simulink model "CCMVgaMotor"  (R2022b)
settings: Solver=FixedStepDiscrete  StartTime=0.0  StopTime=10.0  FixedStep=0.01  RelTol=1e-3
model workspace (MAT data in the model file):
  NvMBlockVgaMtrCalData : Simulink.Signal (value not decoded)
  NvMBlockVgaMtrCalDataInit : Simulink.Parameter (value not decoded)
  VgaWakeUpFlag : Simulink.Signal (value not decoded)
symbols that are objects in the model workspace (value not decoded): NvMBlockVgaMtrCalDataInit
undefined symbols (not defined in the file or in its folder): ComChlType, DioChannelType, LinFrameType, NetworkChlType, NvMBlockId, P_DioLevelType, P_LinIfSchHandleType, P_Std_ReturnType, PowerChlType, VGAChlType
(part 3 of 4)

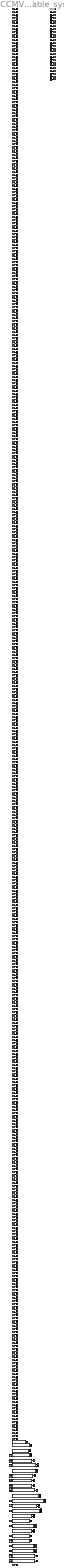
== system CCMVgaMotor/CCMVgaMotor_Runnable_sys ==
  CarCfgForClimaCtrl_CarCfgForAirClng_read : Inport
  CarCfgForClimaCtrl_CarCfgForAirClnrAdv_read : Inport  Port=2
  CarCfgForClimaCtrl_CarCfgForAirFrag_read : Inport  Port=3
  CarCfgForClimaCtrl_CarCfgForAirMtrlSnsr_read : Inport  Port=4
  CarCfgForClimaCtrl_CarCfgForAirQly_read : Inport  Port=5
  CarCfgForClimaCtrl_CarCfgForAmntOfSeat_read : Inport  Port=6
  CarCfgForClimaCtrl_CarCfgForAuxWtrPmp_read : Inport  Port=7
  CarCfgForClimaCtrl_CarCfgForBypVlvInHvac_read : Inport  Port=8
  CarCfgForClimaCtrl_CarCfgForClsVlvInHvac_read : Inport  Port=9
  CarCfgForClimaCtrl_CarCfgForCmprAcTyp_read : Inport  Port=10
  CarCfgForClimaCtrl_CarCfgForCmptmtClng_read : Inport  Port=11
  CarCfgForClimaCtrl_CarCfgForElecVentAdjmt_read : Inport  Port=12
  CarCfgForClimaCtrl_CarCfgForFlcTyp_read : Inport  Port=13
  CarCfgForClimaCtrl_CarCfgForFoldCSD_read : Inport  Port=14
  CarCfgForClimaCtrl_CarCfgForFuTyp_read : Inport  Port=15
  CarCfgForClimaCtrl_CarCfgForGlassTyp_read : Inport  Port=16
  CarCfgForClimaCtrl_CarCfgForHeatPmp_read : Inport  Port=17
  CarCfgForClimaCtrl_CarCfgForHeatrDurgDrvg_read : Inport  Port=18
  CarCfgForClimaCtrl_CarCfgForHeatrTyp_read : Inport  Port=19
  CarCfgForClimaCtrl_CarCfgForHvacFilTyp_read : Inport  Port=20
  CarCfgForClimaCtrl_CarCfgForHvacFlrSnsrForT_read : Inport  Port=21
  CarCfgForClimaCtrl_CarCfgForHvacHeatrSnsrT_read : Inport  Port=22
  CarCfgForClimaCtrl_CarCfgForHvacRe_read : Inport  Port=23
  CarCfgForClimaCtrl_CarCfgForHvacTyp_read : Inport  Port=24
  CarCfgForClimaCtrl_CarCfgForLoVoltElecCfg_read : Inport  Port=25
  CarCfgForClimaCtrl_CarCfgForNavSysandIntnmap_read : Inport  Port=26
  CarCfgForClimaCtrl_CarCfgForNrOfDoor_read : Inport  Port=27
  CarCfgForClimaCtrl_CarCfgForPipeInFuTank_read : Inport  Port=28
  CarCfgForClimaCtrl_CarCfgForPreClng_read : Inport  Port=29
  CarCfgForClimaCtrl_CarCfgForPrkgCDSNotAvl_read : Inport  Port=30
  CarCfgForClimaCtrl_CarCfgForPrkgClima_read : Inport  Port=31
  CarCfgForClimaCtrl_CarCfgForPrpsnTyp_read : Inport  Port=32
  CarCfgForClimaCtrl_CarCfgForRemEngStrtPreCdn_read : Inport  Port=33
  CarCfgForClimaCtrl_CarCfgForRoofHatch_read : Inport  Port=34
  CarCfgForClimaCtrl_CarCfgForSeatHeatgCal_read : Inport  Port=35
  CarCfgForClimaCtrl_CarCfgForSeatHeatgForRowFirst_read : Inport  Port=36
  CarCfgForClimaCtrl_CarCfgForSeatHeatgForRowSec_read : Inport  Port=37
  CarCfgForClimaCtrl_CarCfgForSeatVentnForRowFirst_read : Inport  Port=38
  CarCfgForClimaCtrl_CarCfgForSeatVentnForRowSec_read : Inport  Port=39
  CarCfgForClimaCtrl_CarCfgForSecRowVentnCenter_read : Inport  Port=40
  CarCfgForClimaCtrl_CarCfgForSecRowVentnSide_read : Inport  Port=41
  CarCfgForClimaCtrl_CarCfgForSmallFlowCyc_read : Inport  Port=42
  CarCfgForClimaCtrl_CarCfgForSmkSnsr_read : Inport  Port=43
  CarCfgForClimaCtrl_CarCfgForSnsrForCmptmtT_read : Inport  Port=44
  CarCfgForClimaCtrl_CarCfgForSnsrHumPrsnt_read : Inport  Port=45
  CarCfgForClimaCtrl_CarCfgForSteerWhlHeatg_read : Inport  Port=46
  CarCfgForClimaCtrl_CarCfgForSteerWhlPosn_read : Inport  Port=47
  CarCfgForClimaCtrl_CarCfgForSunSnsr_read : Inport  Port=48
  CarCfgForClimaCtrl_CarCfgForTelmNodTyp_read : Inport  Port=49
  CarCfgForClimaCtrl_CarCfgForThermMngt_read : Inport  Port=50
  CarCfgForClimaCtrl_CarCfgForVehTyp_read : Inport  Port=51
  CarCfgForClimaCtrl_CarCfgForVgaMotorExistSts_read : Inport  Port=52
  CarCfgForClimaCtrl_CarCfgForWindHeatd_read : Inport  Port=53
  CarCfgForClimaCtrl_CarCfgForAutDemst_read : Inport  Port=54
  CarCfgForClimaCtrl_CarCfgForExtVentnClimaCtrl_read : Inport  Port=55
  CarCfgForClimaCtrl_CarCfgForStartDevice_read : Inport  Port=56
  HmiElecAirSwngReq_HmiElecAirSwngReq_read : Inport  Port=57
  LIN2ScheTableReq_Val_read : Inport  Port=58
  SysServCarCfgSts_SysServCarID_read : Inport  Port=59
  SysServCarCfgSts_SysServCCPAQSSts_read : Inport  Port=60
  SysServCarCfgSts_SysServCCPHEXSensorSts_read : Inport  Port=61
  SysServCarCfgSts_SysServCCPLin2AFUSts_read : Inport  Port=62
  SysServCarCfgSts_SysServCCPLin2AIUSts_read : Inport  Port=63
  SysServCarCfgSts_SysServCCPLin2CDSSts_read : Inport  Port=64
  SysServCarCfgSts_SysServCCPLin2PMSISts_read : Inport  Port=65
  SysServCarCfgSts_SysServCCPLin3VGASts_read : Inport  Port=66
  SysServCarCfgSts_SysServDUAirVentSnrSts_read : Inport  Port=67
  SysServCarCfgSts_SysServDUBlowerPwmSignSts_read : Inport  Port=68
  SysServCarCfgSts_SysServDUBlowerPwmSts_read : Inport  Port=69
  SysServCarCfgSts_SysServDUDCDefrostSts_read : Inport  Port=70
  SysServCarCfgSts_SysServDUDCModeSts_read : Inport  Port=71
  SysServCarCfgSts_SysServDUDCRecSts_read : Inport  Port=72
  SysServCarCfgSts_SysServDUDCSModeSts_read : Inport  Port=73
  SysServCarCfgSts_SysServDUDCSTLSts_read : Inport  Port=74
  SysServCarCfgSts_SysServDUDCTLSts_read : Inport  Port=75
  SysServCarCfgSts_SysServDUDCTRSts_read : Inport  Port=76
  SysServCarCfgSts_SysServDUFLFootSts_read : Inport  Port=77
  SysServCarCfgSts_SysServDUFLVentSts_read : Inport  Port=78
  SysServCarCfgSts_SysServDUFRFootSts_read : Inport  Port=79
  SysServCarCfgSts_SysServDUFRVentSts_read : Inport  Port=80
  SysServCarCfgSts_SysServDUIncarMotorSts_read : Inport  Port=81
  SysServCarCfgSts_SysServDULin1HBMFSts_read : Inport  Port=82
  SysServCarCfgSts_SysServDULin4HFAFSts_read : Inport  Port=83
  SysServCarCfgSts_SysServDUSFLFootSts_read : Inport  Port=84
  SysServCarCfgSts_SysServDUSFLVentSts_read : Inport  Port=85
  SysServCarCfgSts_SysServDUSFRFootSts_read : Inport  Port=86
  SysServCarCfgSts_SysServDUSFRVentSts_read : Inport  Port=87
  SysServCarCfgSts_SysServDUSnsrForFCmptmtTSts_read : Inport  Port=88
  SysServCarCfgSts_SysServDUSnsrForRair_read : Inport  Port=89
  SysServCarCfgSts_SysServDUSnsrForRCmptmtTSts_read : Inport  Port=90
  VehModMngtGlbSafe1_VehModMngtGlbSafe1_read : Inport  Port=91
  BatLoadBtrMSts_BatLoadBtrMSts_read : Inport  Port=92
  BatSysBtrMSts_BatSysBtrMSts_read : Inport  Port=93
  VgaMotSetPowerOnInit_PowerOnInit_read : Inport  Port=94
  CarCfgForClimaCtrl_CarCfgForBRAND_read : Inport  Port=95
  CarCfgForClimaCtrl_CarCfgForHeadRadar_read : Inport  Port=96
  CarCfgForClimaCtrl_CarCfgForInteriorViewCamera_read : Inport  Port=97
  CarCfgForClimaCtrl_CarCfgForLifeDetection_read : Inport  Port=98
  CarCfgForClimaCtrl_CarCfgForPowerSeats_read : Inport  Port=99
  CarCfgForClimaCtrl_CarCfgForPTCInFrontHVAC_read : Inport  Port=100
  CarCfgForClimaCtrl_CarCfgForVentLiftMotorExiSts_read : Inport  Port=101
  CarCfgForClimaCtrl_CarCfgForWadingAssistSystem_read : Inport  Port=102
  SbcPwrOn_SbcPwrOn_read : Inport  Port=103
  SbcWakeupSta_SbcWakeupSta_read : Inport  Port=104
  SysServCarCfgSts_SysServCCPBGMNum_read : Inport  Port=105
  SysServCarCfgSts_SysServCCPLin2AQSSts_read : Inport  Port=106
  SysServCarCfgSts_SysServDUBlowerPwmSignTypeSts_read : Inport  Port=107
  SysServCarCfgSts_SysServDUDCFLMdeSts_read : Inport  Port=108
  SysServCarCfgSts_SysServDUReBlowerPwmSignSts_read : Inport  Port=109
  SysServCarCfgSts_SysServDUReBlowerPwmSts_read : Inport  Port=110
  SysServCarCfgSts_SysServLowSideRETXVSts_read : Inport  Port=111
  VentnActr01ActPson_VentnActr01ActPson_read : Inport  Port=112
  VentnActr01ActPson_VentnActr01ActPson_IsUpdated_read : Inport  Port=113
  VentnActr01BlckInd_VentnActr01BlckInd_read : Inport  Port=114
  VentnActr01BlckSts_VentnActr01BlckSts_read : Inport  Port=115
  VentnActr01BusAdr_VentnActr01BusAdr_read : Inport  Port=116
  VentnActr01CoilSts_VentnActr01CoilSts_read : Inport  Port=117
  VentnActr01DrvDir_VentnActr01DrvDir_read : Inport  Port=118
  VentnActr01ElecErr_VentnActr01ElecErr_read : Inport  Port=119
  VentnActr01Mode_VentnActr01Mode_read : Inport  Port=120
  VentnActr01MovDir_VentnActr01MovDir_read : Inport  Port=121
  VentnActr01OvrTempErr_VentnActr01OvrTempErr_read : Inport  Port=122
  VentnActr01PsonSts_VentnActr01PsonSts_read : Inport  Port=123
  VentnActr01ResetSts_VentnActr01ResetSts_read : Inport  Port=124
  VentnActr01SpclFct_VentnActr01SpclFct_read : Inport  Port=125
  VentnActr01SpdSts_VentnActr01SpdSts_read : Inport  Port=126
  VentnActr01TrqSts_VentnActr01TrqSts_read : Inport  Port=127
  VentnActr01UrgcExist_VentnActr01UrgcExist_read : Inport  Port=128
  VentnActr01UrgcPson_VentnActr01UrgcPson_read : Inport  Port=129
  VentnActr01UrgcSts_VentnActr01UrgcSts_read : Inport  Port=130
  VentnActr01VoltErr_VentnActr01VoltErr_read : Inport  Port=131
  VentnActr02ActPson_VentnActr02ActPson_read : Inport  Port=132
  VentnActr02ActPson_VentnActr02ActPson_IsUpdated_read : Inport  Port=133
  VentnActr02BlckInd_VentnActr02BlckInd_read : Inport  Port=134
  VentnActr02BlckSts_VentnActr02BlckSts_read : Inport  Port=135
  VentnActr02BusAdr_VentnActr02BusAdr_read : Inport  Port=136
  VentnActr02CoilSts_VentnActr02CoilSts_read : Inport  Port=137
  VentnActr02DrvDir_VentnActr02DrvDir_read : Inport  Port=138
  VentnActr02ElecErr_VentnActr02ElecErr_read : Inport  Port=139
  VentnActr02Mode_VentnActr02Mode_read : Inport  Port=140
  VentnActr02MovDir_VentnActr02MovDir_read : Inport  Port=141
  VentnActr02OvrTempErr_VentnActr02OvrTempErr_read : Inport  Port=142
  VentnActr02PsonSts_VentnActr02PsonSts_read : Inport  Port=143
  VentnActr02ResetSts_VentnActr02ResetSts_read : Inport  Port=144
  VentnActr02SpclFct_VentnActr02SpclFct_read : Inport  Port=145
  VentnActr02SpdSts_VentnActr02SpdSts_read : Inport  Port=146
  VentnActr02TrqSts_VentnActr02TrqSts_read : Inport  Port=147
  VentnActr02UrgcExist_VentnActr02UrgcExist_read : Inport  Port=148
  VentnActr02UrgcPson_VentnActr02UrgcPson_read : Inport  Port=149
  VentnActr02UrgcSts_VentnActr02UrgcSts_read : Inport  Port=150
  VentnActr02VoltErr_VentnActr02VoltErr_read : Inport  Port=151
  VentnActr03ActPson_VentnActr03ActPson_read : Inport  Port=152
  VentnActr03ActPson_VentnActr03ActPson_IsUpdated_read : Inport  Port=153
  VentnActr03BlckInd_VentnActr03BlckInd_read : Inport  Port=154
  VentnActr03BlckSts_VentnActr03BlckSts_read : Inport  Port=155
  VentnActr03BusAdr_VentnActr03BusAdr_read : Inport  Port=156
  VentnActr03CoilSts_VentnActr03CoilSts_read : Inport  Port=157
  VentnActr03DrvDir_VentnActr03DrvDir_read : Inport  Port=158
  VentnActr03ElecErr_VentnActr03ElecErr_read : Inport  Port=159
  VentnActr03Mode_VentnActr03Mode_read : Inport  Port=160
  VentnActr03MovDir_VentnActr03MovDir_read : Inport  Port=161
  VentnActr03OvrTempErr_VentnActr03OvrTempErr_read : Inport  Port=162
  VentnActr03PsonSts_VentnActr03PsonSts_read : Inport  Port=163
  VentnActr03ResetSts_VentnActr03ResetSts_read : Inport  Port=164
  VentnActr03SpclFct_VentnActr03SpclFct_read : Inport  Port=165
  VentnActr03SpdSts_VentnActr03SpdSts_read : Inport  Port=166
  VentnActr03TrqSts_VentnActr03TrqSts_read : Inport  Port=167
  VentnActr03UrgcExist_VentnActr03UrgcExist_read : Inport  Port=168
  VentnActr03UrgcPson_VentnActr03UrgcPson_read : Inport  Port=169
  VentnActr03UrgcSts_VentnActr03UrgcSts_read : Inport  Port=170
  VentnActr03VoltErr_VentnActr03VoltErr_read : Inport  Port=171
  VentnActr04ActPson_VentnActr04ActPson_read : Inport  Port=172
  VentnActr04ActPson_VentnActr04ActPson_IsUpdated_read : Inport  Port=173
  VentnActr04BlckInd_VentnActr04BlckInd_read : Inport  Port=174
  VentnActr04BlckSts_VentnActr04BlckSts_read : Inport  Port=175
  VentnActr04BusAdr_VentnActr04BusAdr_read : Inport  Port=176
  VentnActr04CoilSts_VentnActr04CoilSts_read : Inport  Port=177
  VentnActr04DrvDir_VentnActr04DrvDir_read : Inport  Port=178
  VentnActr04ElecErr_VentnActr04ElecErr_read : Inport  Port=179
  VentnActr04Mode_VentnActr04Mode_read : Inport  Port=180
  VentnActr04MovDir_VentnActr04MovDir_read : Inport  Port=181
  VentnActr04OvrTempErr_VentnActr04OvrTempErr_read : Inport  Port=182
  VentnActr04PsonSts_VentnActr04PsonSts_read : Inport  Port=183
  VentnActr04ResetSts_VentnActr04ResetSts_read : Inport  Port=184
  VentnActr04SpclFct_VentnActr04SpclFct_read : Inport  Port=185
  VentnActr04SpdSts_VentnActr04SpdSts_read : Inport  Port=186
  VentnActr04TrqSts_VentnActr04TrqSts_read : Inport  Port=187
  VentnActr04UrgcExist_VentnActr04UrgcExist_read : Inport  Port=188
  VentnActr04UrgcPson_VentnActr04UrgcPson_read : Inport  Port=189
  VentnActr04UrgcSts_VentnActr04UrgcSts_read : Inport  Port=190
  VentnActr04VoltErr_VentnActr04VoltErr_read : Inport  Port=191
  VentnActr05ActPson_VentnActr05ActPson_read : Inport  Port=192
  VentnActr05ActPson_VentnActr05ActPson_IsUpdated_read : Inport  Port=193
  VentnActr05BlckInd_VentnActr05BlckInd_read : Inport  Port=194
  VentnActr05BlckSts_VentnActr05BlckSts_read : Inport  Port=195
  VentnActr05BusAdr_VentnActr05BusAdr_read : Inport  Port=196
  VentnActr05CoilSts_VentnActr05CoilSts_read : Inport  Port=197
  VentnActr05DrvDir_VentnActr05DrvDir_read : Inport  Port=198
  VentnActr05ElecErr_VentnActr05ElecErr_read : Inport  Port=199
  VentnActr05Mode_VentnActr05Mode_read : Inport  Port=200
  VentnActr05MovDir_VentnActr05MovDir_read : Inport  Port=201
  VentnActr05OvrTempErr_VentnActr05OvrTempErr_read : Inport  Port=202
  VentnActr05PsonSts_VentnActr05PsonSts_read : Inport  Port=203
  VentnActr05ResetSts_VentnActr05ResetSts_read : Inport  Port=204
  VentnActr05SpclFct_VentnActr05SpclFct_read : Inport  Port=205
  VentnActr05SpdSts_VentnActr05SpdSts_read : Inport  Port=206
  VentnActr05TrqSts_VentnActr05TrqSts_read : Inport  Port=207
  VentnActr05UrgcExist_VentnActr05UrgcExist_read : Inport  Port=208
  VentnActr05UrgcPson_VentnActr05UrgcPson_read : Inport  Port=209
  VentnActr05UrgcSts_VentnActr05UrgcSts_read : Inport  Port=210
  VentnActr05VoltErr_VentnActr05VoltErr_read : Inport  Port=211
  VentnActr06ActPson_VentnActr06ActPson_read : Inport  Port=212
  VentnActr06ActPson_VentnActr06ActPson_IsUpdated_read : Inport  Port=213
  VentnActr06BlckInd_VentnActr06BlckInd_read : Inport  Port=214
  VentnActr06BlckSts_VentnActr06BlckSts_read : Inport  Port=215
  VentnActr06BusAdr_VentnActr06BusAdr_read : Inport  Port=216
  VentnActr06CoilSts_VentnActr06CoilSts_read : Inport  Port=217
  VentnActr06DrvDir_VentnActr06DrvDir_read : Inport  Port=218
  VentnActr06ElecErr_VentnActr06ElecErr_read : Inport  Port=219
  VentnActr06Mode_VentnActr06Mode_read : Inport  Port=220
  VentnActr06MovDir_VentnActr06MovDir_read : Inport  Port=221
  VentnActr06OvrTempErr_VentnActr06OvrTempErr_read : Inport  Port=222
  VentnActr06PsonSts_VentnActr06PsonSts_read : Inport  Port=223
  VentnActr06ResetSts_VentnActr06ResetSts_read : Inport  Port=224
  VentnActr06SpclFct_VentnActr06SpclFct_read : Inport  Port=225
  VentnActr06SpdSts_VentnActr06SpdSts_read : Inport  Port=226
  VentnActr06TrqSts_VentnActr06TrqSts_read : Inport  Port=227
  VentnActr06UrgcExist_VentnActr06UrgcExist_read : Inport  Port=228
  VentnActr06UrgcPson_VentnActr06UrgcPson_read : Inport  Port=229
  VentnActr06UrgcSts_VentnActr06UrgcSts_read : Inport  Port=230
  VentnActr06VoltErr_VentnActr06VoltErr_read : Inport  Port=231
  VentnActr07ActPson_VentnActr07ActPson_read : Inport  Port=232
  VentnActr07ActPson_VentnActr07ActPson_IsUpdated_read : Inport  Port=233
  VentnActr07BlckInd_VentnActr07BlckInd_read : Inport  Port=234
  VentnActr07BlckSts_VentnActr07BlckSts_read : Inport  Port=235
  VentnActr07BusAdr_VentnActr07BusAdr_read : Inport  Port=236
  VentnActr07CoilSts_VentnActr07CoilSts_read : Inport  Port=237
  VentnActr07DrvDir_VentnActr07DrvDir_read : Inport  Port=238
  VentnActr07ElecErr_VentnActr07ElecErr_read : Inport  Port=239
  VentnActr07Mode_VentnActr07Mode_read : Inport  Port=240
  VentnActr07MovDir_VentnActr07MovDir_read : Inport  Port=241
  VentnActr07OvrTempErr_VentnActr07OvrTempErr_read : Inport  Port=242
  VentnActr07PsonSts_VentnActr07PsonSts_read : Inport  Port=243
  VentnActr07ResetSts_VentnActr07ResetSts_read : Inport  Port=244
  VentnActr07SpclFct_VentnActr07SpclFct_read : Inport  Port=245
  VentnActr07SpdSts_VentnActr07SpdSts_read : Inport  Port=246
  VentnActr07TrqSts_VentnActr07TrqSts_read : Inport  Port=247
  VentnActr07UrgcExist_VentnActr07UrgcExist_read : Inport  Port=248
  VentnActr07UrgcPson_VentnActr07UrgcPson_read : Inport  Port=249
  VentnActr07UrgcSts_VentnActr07UrgcSts_read : Inport  Port=250
  VentnActr07VoltErr_VentnActr07VoltErr_read : Inport  Port=251
  VentnActr08ActPson_VentnActr08ActPson_read : Inport  Port=252
  VentnActr08ActPson_VentnActr08ActPson_IsUpdated_read : Inport  Port=253
  VentnActr08BlckInd_VentnActr08BlckInd_read : Inport  Port=254
  VentnActr08BlckSts_VentnActr08BlckSts_read : Inport  Port=255
  VentnActr08BusAdr_VentnActr08BusAdr_read : Inport  Port=256
  VentnActr08CoilSts_VentnActr08CoilSts_read : Inport  Port=257
  VentnActr08DrvDir_VentnActr08DrvDir_read : Inport  Port=258
  VentnActr08ElecErr_VentnActr08ElecErr_read : Inport  Port=259
  VentnActr08Mode_VentnActr08Mode_read : Inport  Port=260
  VentnActr08MovDir_VentnActr08MovDir_read : Inport  Port=261
  VentnActr08OvrTempErr_VentnActr08OvrTempErr_read : Inport  Port=262
  VentnActr08PsonSts_VentnActr08PsonSts_read : Inport  Port=263
  VentnActr08ResetSts_VentnActr08ResetSts_read : Inport  Port=264
  VentnActr08SpclFct_VentnActr08SpclFct_read : Inport  Port=265
  VentnActr08SpdSts_VentnActr08SpdSts_read : Inport  Port=266
  VentnActr08TrqSts_VentnActr08TrqSts_read : Inport  Port=267
  VentnActr08UrgcExist_VentnActr08UrgcExist_read : Inport  Port=268
  VentnActr08UrgcPson_VentnActr08UrgcPson_read : Inport  Port=269
  VentnActr08UrgcSts_VentnActr08UrgcSts_read : Inport  Port=270
  VentnActr08VoltErr_VentnActr08VoltErr_read : Inport  Port=271
  VentnActr09ActPson_VentnActr09ActPson_read : Inport  Port=272
  VentnActr09ActPson_VentnActr09ActPson_IsUpdated_read : Inport  Port=273
  VentnActr09BlckInd_VentnActr09BlckInd_read : Inport  Port=274
  VentnActr09BlckSts_VentnActr09BlckSts_read : Inport  Port=275
  VentnActr09BusAdr_VentnActr09BusAdr_read : Inport  Port=276
  VentnActr09CoilSts_VentnActr09CoilSts_read : Inport  Port=277
  VentnActr09DrvDir_VentnActr09DrvDir_read : Inport  Port=278
  VentnActr09ElecErr_VentnActr09ElecErr_read : Inport  Port=279
  VentnActr09Mode_VentnActr09Mode_read : Inport  Port=280
  VentnActr09MovDir_VentnActr09MovDir_read : Inport  Port=281
  VentnActr09OvrTempErr_VentnActr09OvrTempErr_read : Inport  Port=282
  VentnActr09PsonSts_VentnActr09PsonSts_read : Inport  Port=283
  VentnActr09ResetSts_VentnActr09ResetSts_read : Inport  Port=284
  VentnActr09SpclFct_VentnActr09SpclFct_read : Inport  Port=285
  VentnActr09SpdSts_VentnActr09SpdSts_read : Inport  Port=286
  VentnActr09TrqSts_VentnActr09TrqSts_read : Inport  Port=287
  VentnActr09UrgcExist_VentnActr09UrgcExist_read : Inport  Port=288
  VentnActr09UrgcPson_VentnActr09UrgcPson_read : Inport  Port=289
  VentnActr09UrgcSts_VentnActr09UrgcSts_read : Inport  Port=290
  VentnActr09VoltErr_VentnActr09VoltErr_read : Inport  Port=291
  VentnActr10ActPson_VentnActr10ActPson_read : Inport  Port=292
  VentnActr10ActPson_VentnActr10ActPson_IsUpdated_read : Inport  Port=293
  VentnActr10BlckInd_VentnActr10BlckInd_read : Inport  Port=294
  VentnActr10BlckSts_VentnActr10BlckSts_read : Inport  Port=295
  VentnActr10BusAdr_VentnActr10BusAdr_read : Inport  Port=296
  VentnActr10CoilSts_VentnActr10CoilSts_read : Inport  Port=297
  VentnActr10DrvDir_VentnActr10DrvDir_read : Inport  Port=298
  VentnActr10ElecErr_VentnActr10ElecErr_read : Inport  Port=299
  VentnActr10Mode_VentnActr10Mode_read : Inport  Port=300
  VentnActr10MovDir_VentnActr10MovDir_read : Inport  Port=301
  VentnActr10OvrTempErr_VentnActr10OvrTempErr_read : Inport  Port=302
  VentnActr10PsonSts_VentnActr10PsonSts_read : Inport  Port=303
  VentnActr10ResetSts_VentnActr10ResetSts_read : Inport  Port=304
  VentnActr10SpclFct_VentnActr10SpclFct_read : Inport  Port=305
  VentnActr10SpdSts_VentnActr10SpdSts_read : Inport  Port=306
  VentnActr10TrqSts_VentnActr10TrqSts_read : Inport  Port=307
  VentnActr10UrgcExist_VentnActr10UrgcExist_read : Inport  Port=308
  VentnActr10UrgcPson_VentnActr10UrgcPson_read : Inport  Port=309
  VentnActr10UrgcSts_VentnActr10UrgcSts_read : Inport  Port=310
  VentnActr10VoltErr_VentnActr10VoltErr_read : Inport  Port=311
  VentnActr11ActPson_VentnActr11ActPson_read : Inport  Port=312
  VentnActr11ActPson_VentnActr11ActPson_IsUpdated_read : Inport  Port=313
  VentnActr11BlckInd_VentnActr11BlckInd_read : Inport  Port=314
  VentnActr11BlckSts_VentnActr11BlckSts_read : Inport  Port=315
  VentnActr11BusAdr_VentnActr11BusAdr_read : Inport  Port=316
  VentnActr11CoilSts_VentnActr11CoilSts_read : Inport  Port=317
  VentnActr11DrvDir_VentnActr11DrvDir_read : Inport  Port=318
  VentnActr11ElecErr_VentnActr11ElecErr_read : Inport  Port=319
  VentnActr11Mode_VentnActr11Mode_read : Inport  Port=320
  VentnActr11MovDir_VentnActr11MovDir_read : Inport  Port=321
  VentnActr11OvrTempErr_VentnActr11OvrTempErr_read : Inport  Port=322
  VentnActr11PsonSts_VentnActr11PsonSts_read : Inport  Port=323
  VentnActr11ResetSts_VentnActr11ResetSts_read : Inport  Port=324
  VentnActr11SpclFct_VentnActr11SpclFct_read : Inport  Port=325
  VentnActr11SpdSts_VentnActr11SpdSts_read : Inport  Port=326
  VentnActr11TrqSts_VentnActr11TrqSts_read : Inport  Port=327
  VentnActr11UrgcExist_VentnActr11UrgcExist_read : Inport  Port=328
  VentnActr11UrgcPson_VentnActr11UrgcPson_read : Inport  Port=329
  VentnActr11UrgcSts_VentnActr11UrgcSts_read : Inport  Port=330
  VentnActr11VoltErr_VentnActr11VoltErr_read : Inport  Port=331
  VentnActr12ActPson_VentnActr12ActPson_read : Inport  Port=332
  VentnActr12ActPson_VentnActr12ActPson_IsUpdated_read : Inport  Port=333
  VentnActr12BlckInd_VentnActr12BlckInd_read : Inport  Port=334
  VentnActr12BlckSts_VentnActr12BlckSts_read : Inport  Port=335
  VentnActr12BusAdr_VentnActr12BusAdr_read : Inport  Port=336
  VentnActr12CoilSts_VentnActr12CoilSts_read : Inport  Port=337
  VentnActr12DrvDir_VentnActr12DrvDir_read : Inport  Port=338
  VentnActr12ElecErr_VentnActr12ElecErr_read : Inport  Port=339
  VentnActr12Mode_VentnActr12Mode_read : Inport  Port=340
  VentnActr12MovDir_VentnActr12MovDir_read : Inport  Port=341
  VentnActr12OvrTempErr_VentnActr12OvrTempErr_read : Inport  Port=342
  VentnActr12PsonSts_VentnActr12PsonSts_read : Inport  Port=343
  VentnActr12ResetSts_VentnActr12ResetSts_read : Inport  Port=344
  VentnActr12SpclFct_VentnActr12SpclFct_read : Inport  Port=345
  VentnActr12SpdSts_VentnActr12SpdSts_read : Inport  Port=346
  VentnActr12TrqSts_VentnActr12TrqSts_read : Inport  Port=347
  VentnActr12UrgcExist_VentnActr12UrgcExist_read : Inport  Port=348
  VentnActr12UrgcPson_VentnActr12UrgcPson_read : Inport  Port=349
  VentnActr12UrgcSts_VentnActr12UrgcSts_read : Inport  Port=350
  VentnActr12VoltErr_VentnActr12VoltErr_read : Inport  Port=351
  VentnActr13ActPson_VentnActr13ActPson_read : Inport  Port=352
  VentnActr13ActPson_VentnActr13ActPson_IsUpdated_read : Inport  Port=353
  VentnActr13BlckInd_VentnActr13BlckInd_read : Inport  Port=354
  VentnActr13BlckSts_VentnActr13BlckSts_read : Inport  Port=355
  VentnActr13BusAdr_VentnActr13BusAdr_read : Inport  Port=356
  VentnActr13CoilSts_VentnActr13CoilSts_read : Inport  Port=357
  VentnActr13DrvDir_VentnActr13DrvDir_read : Inport  Port=358
  VentnActr13ElecErr_VentnActr13ElecErr_read : Inport  Port=359
  VentnActr13Mode_VentnActr13Mode_read : Inport  Port=360
  VentnActr13MovDir_VentnActr13MovDir_read : Inport  Port=361
  VentnActr13OvrTempErr_VentnActr13OvrTempErr_read : Inport  Port=362
  VentnActr13PsonSts_VentnActr13PsonSts_read : Inport  Port=363
  VentnActr13ResetSts_VentnActr13ResetSts_read : Inport  Port=364
  VentnActr13SpclFct_VentnActr13SpclFct_read : Inport  Port=365
  VentnActr13SpdSts_VentnActr13SpdSts_read : Inport  Port=366
  VentnActr13TrqSts_VentnActr13TrqSts_read : Inport  Port=367
  VentnActr13UrgcExist_VentnActr13UrgcExist_read : Inport  Port=368
  VentnActr13UrgcPson_VentnActr13UrgcPson_read : Inport  Port=369
  VentnActr13UrgcSts_VentnActr13UrgcSts_read : Inport  Port=370
  VentnActr13VoltErr_VentnActr13VoltErr_read : Inport  Port=371
  VentnActr14ActPson_VentnActr14ActPson_read : Inport  Port=372
  VentnActr14ActPson_VentnActr14ActPson_IsUpdated_read : Inport  Port=373
  VentnActr14BlckInd_VentnActr14BlckInd_read : Inport  Port=374
  VentnActr14BlckSts_VentnActr14BlckSts_read : Inport  Port=375
  VentnActr14BusAdr_VentnActr14BusAdr_read : Inport  Port=376
  VentnActr14CoilSts_VentnActr14CoilSts_read : Inport  Port=377
  VentnActr14DrvDir_VentnActr14DrvDir_read : Inport  Port=378
  VentnActr14ElecErr_VentnActr14ElecErr_read : Inport  Port=379
  VentnActr14Mode_VentnActr14Mode_read : Inport  Port=380
  VentnActr14MovDir_VentnActr14MovDir_read : Inport  Port=381
  VentnActr14OvrTempErr_VentnActr14OvrTempErr_read : Inport  Port=382
  VentnActr14PsonSts_VentnActr14PsonSts_read : Inport  Port=383
  VentnActr14ResetSts_VentnActr14ResetSts_read : Inport  Port=384
  VentnActr14SpclFct_VentnActr14SpclFct_read : Inport  Port=385
  VentnActr14SpdSts_VentnActr14SpdSts_read : Inport  Port=386
  VentnActr14TrqSts_VentnActr14TrqSts_read : Inport  Port=387
  VentnActr14UrgcExist_VentnActr14UrgcExist_read : Inport  Port=388
  VentnActr14UrgcPson_VentnActr14UrgcPson_read : Inport  Port=389
  VentnActr14UrgcSts_VentnActr14UrgcSts_read : Inport  Port=390
  VentnActr14VoltErr_VentnActr14VoltErr_read : Inport  Port=391
  VentnActr15ActPson_VentnActr15ActPson_read : Inport  Port=392
  VentnActr15ActPson_VentnActr15ActPson_IsUpdated_read : Inport  Port=393
  VentnActr15BlckInd_VentnActr15BlckInd_read : Inport  Port=394
  VentnActr15BlckSts_VentnActr15BlckSts_read : Inport  Port=395
  VentnActr15BusAdr_VentnActr15BusAdr_read : Inport  Port=396
  VentnActr15CoilSts_VentnActr15CoilSts_read : Inport  Port=397
  VentnActr15DrvDir_VentnActr15DrvDir_read : Inport  Port=398
  VentnActr15ElecErr_VentnActr15ElecErr_read : Inport  Port=399
  VentnActr15Mode_VentnActr15Mode_read : Inport  Port=400
  VentnActr15MovDir_VentnActr15MovDir_read : Inport  Port=401
  VentnActr15OvrTempErr_VentnActr15OvrTempErr_read : Inport  Port=402
  VentnActr15PsonSts_VentnActr15PsonSts_read : Inport  Port=403
  VentnActr15ResetSts_VentnActr15ResetSts_read : Inport  Port=404
  VentnActr15SpclFct_VentnActr15SpclFct_read : Inport  Port=405
  VentnActr15SpdSts_VentnActr15SpdSts_read : Inport  Port=406
  VentnActr15TrqSts_VentnActr15TrqSts_read : Inport  Port=407
  VentnActr15UrgcExist_VentnActr15UrgcExist_read : Inport  Port=408
  VentnActr15UrgcPson_VentnActr15UrgcPson_read : Inport  Port=409
  VentnActr15UrgcSts_VentnActr15UrgcSts_read : Inport  Port=410
  VentnActr15VoltErr_VentnActr15VoltErr_read : Inport  Port=411
  VentnActr16ActPson_VentnActr16ActPson_read : Inport  Port=412
  VentnActr16BlckInd_VentnActr16BlckInd_read : Inport  Port=413
  VentnActr16BlckSts_VentnActr16BlckSts_read : Inport  Port=414
  VentnActr16BusAdr_VentnActr16BusAdr_read : Inport  Port=415
  VentnActr16CoilSts_VentnActr16CoilSts_read : Inport  Port=416
  VentnActr16DrvDir_VentnActr16DrvDir_read : Inport  Port=417
  VentnActr16ElecErr_VentnActr16ElecErr_read : Inport  Port=418
  VentnActr16Mode_VentnActr16Mode_read : Inport  Port=419
  VentnActr16MovDir_VentnActr16MovDir_read : Inport  Port=420
  VentnActr16OvrTempErr_VentnActr16OvrTempErr_read : Inport  Port=421
  VentnActr16PsonSts_VentnActr16PsonSts_read : Inport  Port=422
  VentnActr16ResetSts_VentnActr16ResetSts_read : Inport  Port=423
  VentnActr16SpclFct_VentnActr16SpclFct_read : Inport  Port=424
  VentnActr16SpdSts_VentnActr16SpdSts_read : Inport  Port=425
  VentnActr16TrqSts_VentnActr16TrqSts_read : Inport  Port=426
  VentnActr16UrgcExist_VentnActr16UrgcExist_read : Inport  Port=427
  VentnActr16UrgcPson_VentnActr16UrgcPson_read : Inport  Port=428
  VentnActr16UrgcSts_VentnActr16UrgcSts_read : Inport  Port=429
  VentnActr16VoltErr_VentnActr16VoltErr_read : Inport  Port=430
  VentnActr17ActPson_VentnActr17ActPson_read : Inport  Port=431
  VentnActr17ActPson_VentnActr17ActPson_IsUpdated_read : Inport  Port=432
  VentnActr17BlckInd_VentnActr17BlckInd_read : Inport  Port=433
  VentnActr17BlckSts_VentnActr17BlckSts_read : Inport  Port=434
  VentnActr17BusAdr_VentnActr17BusAdr_read : Inport  Port=435
  VentnActr17CoilSts_VentnActr17CoilSts_read : Inport  Port=436
  VentnActr17DrvDir_VentnActr17DrvDir_read : Inport  Port=437
  VentnActr17ElecErr_VentnActr17ElecErr_read : Inport  Port=438
  VentnActr17Mode_VentnActr17Mode_read : Inport  Port=439
  VentnActr17MovDir_VentnActr17MovDir_read : Inport  Port=440
  VentnActr17OvrTempErr_VentnActr17OvrTempErr_read : Inport  Port=441
  VentnActr17PsonSts_VentnActr17PsonSts_read : Inport  Port=442
  VentnActr17ResetSts_VentnActr17ResetSts_read : Inport  Port=443
  VentnActr17SpclFct_VentnActr17SpclFct_read : Inport  Port=444
  VentnActr17SpdSts_VentnActr17SpdSts_read : Inport  Port=445
  VentnActr17TrqSts_VentnActr17TrqSts_read : Inport  Port=446
  VentnActr17UrgcExist_VentnActr17UrgcExist_read : Inport  Port=447
  VentnActr17UrgcPson_VentnActr17UrgcPson_read : Inport  Port=448
  VentnActr17UrgcSts_VentnActr17UrgcSts_read : Inport  Port=449
  VentnActr17VoltErr_VentnActr17VoltErr_read : Inport  Port=450
  VentnActr18ActPson_VentnActr18ActPson_read : Inport  Port=451
  VentnActr18BlckInd_VentnActr18BlckInd_read : Inport  Port=452
  VentnActr18BlckSts_VentnActr18BlckSts_read : Inport  Port=453
  VentnActr18BusAdr_VentnActr18BusAdr_read : Inport  Port=454
  VentnActr18CoilSts_VentnActr18CoilSts_read : Inport  Port=455
  VentnActr18DrvDir_VentnActr18DrvDir_read : Inport  Port=456
  VentnActr18ElecErr_VentnActr18ElecErr_read : Inport  Port=457
  VentnActr18Mode_VentnActr18Mode_read : Inport  Port=458
  VentnActr18MovDir_VentnActr18MovDir_read : Inport  Port=459
  VentnActr18OvrTempErr_VentnActr18OvrTempErr_read : Inport  Port=460
  VentnActr18PsonSts_VentnActr18PsonSts_read : Inport  Port=461
  VentnActr18ResetSts_VentnActr18ResetSts_read : Inport  Port=462
  VentnActr18SpclFct_VentnActr18SpclFct_read : Inport  Port=463
  VentnActr18SpdSts_VentnActr18SpdSts_read : Inport  Port=464
  VentnActr18TrqSts_VentnActr18TrqSts_read : Inport  Port=465
  VentnActr18UrgcExist_VentnActr18UrgcExist_read : Inport  Port=466
  VentnActr18UrgcPson_VentnActr18UrgcPson_read : Inport  Port=467
  VentnActr18UrgcSts_VentnActr18UrgcSts_read : Inport  Port=468
  VentnActr18VoltErr_VentnActr18VoltErr_read : Inport  Port=469
  VentnActr19ActPson_VentnActr19ActPson_read : Inport  Port=470
  VentnActr19ActPson_VentnActr19ActPson_IsUpdated_read : Inport  Port=471
  VentnActr19BlckInd_VentnActr19BlckInd_read : Inport  Port=472
  VentnActr19BlckSts_VentnActr19BlckSts_read : Inport  Port=473
  VentnActr19BusAdr_VentnActr19BusAdr_read : Inport  Port=474
  VentnActr19CoilSts_VentnActr19CoilSts_read : Inport  Port=475
  VentnActr19DrvDir_VentnActr19DrvDir_read : Inport  Port=476
  VentnActr19ElecErr_VentnActr19ElecErr_read : Inport  Port=477
  VentnActr19Mode_VentnActr19Mode_read : Inport  Port=478
  VentnActr19MovDir_VentnActr19MovDir_read : Inport  Port=479
  VentnActr19OvrTempErr_VentnActr19OvrTempErr_read : Inport  Port=480
  VentnActr19PsonSts_VentnActr19PsonSts_read : Inport  Port=481
  VentnActr19ResetSts_VentnActr19ResetSts_read : Inport  Port=482
  VentnActr19SpclFct_VentnActr19SpclFct_read : Inport  Port=483
  VentnActr19SpdSts_VentnActr19SpdSts_read : Inport  Port=484
  VentnActr19TrqSts_VentnActr19TrqSts_read : Inport  Port=485
  VentnActr19UrgcExist_VentnActr19UrgcExist_read : Inport  Port=486
  VentnActr19UrgcPson_VentnActr19UrgcPson_read : Inport  Port=487
  VentnActr19UrgcSts_VentnActr19UrgcSts_read : Inport  Port=488
  VentnActr19VoltErr_VentnActr19VoltErr_read : Inport  Port=489
  VentnActr20ActPson_VentnActr20ActPson_read : Inport  Port=490
  VentnActr20ActPson_VentnActr20ActPson_IsUpdated_read : Inport  Port=491
  VentnActr20BlckInd_VentnActr20BlckInd_read : Inport  Port=492
  VentnActr20BlckSts_VentnActr20BlckSts_read : Inport  Port=493
  VentnActr20BusAdr_VentnActr20BusAdr_read : Inport  Port=494
  VentnActr20CoilSts_VentnActr20CoilSts_read : Inport  Port=495
  VentnActr20DrvDir_VentnActr20DrvDir_read : Inport  Port=496
  VentnActr20ElecErr_VentnActr20ElecErr_read : Inport  Port=497
  VentnActr20Mode_VentnActr20Mode_read : Inport  Port=498
  VentnActr20MovDir_VentnActr20MovDir_read : Inport  Port=499
  VentnActr20OvrTempErr_VentnActr20OvrTempErr_read : Inport  Port=500
  VentnActr20PsonSts_VentnActr20PsonSts_read : Inport  Port=501
  VentnActr20ResetSts_VentnActr20ResetSts_read : Inport  Port=502
  VentnActr20SpclFct_VentnActr20SpclFct_read : Inport  Port=503
  VentnActr20SpdSts_VentnActr20SpdSts_read : Inport  Port=504
  VentnActr20TrqSts_VentnActr20TrqSts_read : Inport  Port=505
  VentnActr20UrgcExist_VentnActr20UrgcExist_read : Inport  Port=506
  VentnActr20UrgcPson_VentnActr20UrgcPson_read : Inport  Port=507
  VentnActr20UrgcSts_VentnActr20UrgcSts_read : Inport  Port=508
  VentnActr20VoltErr_VentnActr20VoltErr_read : Inport  Port=509
  VGAStopDuePowerLimit_VGAStopDuePowerLimit_read : Inport  Port=510
  BswLifeCycle_LifeCycle_read : Inport  Port=511
  function : TriggerPort  TriggerType=function-call  VariantControl=(inherit)  SampleTimeType=periodic  SampleTime=-1
  DioServ_PowerOffInitChannel : FunctionCaller  FunctionPrototype=ERR = DioServ_PowerOffInitChannel()  OutputArgumentSpecifications=P_Std_ReturnType
  DioServ_PowerOnInitChannel : FunctionCaller  FunctionPrototype=ERR = DioServ_PowerOnInitChannel()  OutputArgumentSpecifications=P_Std_ReturnType
  DioServ_ReadChannel : FunctionCaller  FunctionPrototype=[Level,ERR] = DioServ_ReadChannel(ChannelId)  InputArgumentSpecifications=DioChannelType(0)  OutputArgumentSpecifications=P_DioLevelType, P_Std_ReturnType
  DioServ_WriteChannel : FunctionCaller  FunctionPrototype=ERR = DioServ_WriteChannel(ChannelId,Level)  InputArgumentSpecifications=DioChannelType(0), P_DioLevelType  OutputArgumentSpecifications=P_Std_ReturnType
  Ground_{1..52} (52 alike) : Ground
  LinIfServ_GetScheduleInfo : FunctionCaller  FunctionPrototype=[ScheduleInfo,ERR] = LinIfServ_GetScheduleInfo(Channel)  InputArgumentSpecifications=NetworkChlType(0)  OutputArgumentSpecifications=P_LinIfScheduleInfoType, P_Std_ReturnType
  LinSMServ_ScheduleRequest : FunctionCaller  FunctionPrototype=ERR = LinSMServ_ScheduleRequest(network,schedule)  InputArgumentSpecifications=NetworkChlType(0), P_LinIfSchHandleType  OutputArgumentSpecifications=P_Std_ReturnType
  LinService_GetLin2WakeupStatus : FunctionCaller  FunctionPrototype=[WakSta,ERR] = LinService_GetLin2WakeupStatus()  OutputArgumentSpecifications=uint8(1), P_Std_ReturnType
  LinService_SendLin3dFrame : FunctionCaller  FunctionPrototype=ERR = LinService_SendLin3dFrame(channel,data)  InputArgumentSpecifications=ComChlType(0), uint8([1])  OutputArgumentSpecifications=P_Std_ReturnType
  LinService_SetLin3cFrame : FunctionCaller  FunctionPrototype=ERR = LinService_SetLin3cFrame(Channel,Data)  InputArgumentSpecifications=ComChlType(0), uint8([1])  OutputArgumentSpecifications=P_Std_ReturnType
  LinService_SetLinTable : FunctionCaller  FunctionPrototype=ERR = LinService_SetLinTable(Channel,Table)  InputArgumentSpecifications=ComChlType(0), uint8(1)  OutputArgumentSpecifications=P_Std_ReturnType
  MirrorLin3ErrServ_GetLinBusTxErrSta : FunctionCaller  FunctionPrototype=[ErrFlag,ERR] = MirrorLin3ErrServ_GetLinBusTxErrSta()  OutputArgumentSpecifications=uint8(1), P_Std_ReturnType
  MirrorLin3ErrServ_GetVgaCcmLin3VgaFr01NoResp : FunctionCaller  FunctionPrototype=[ErrFlag,ERR] = MirrorLin3ErrServ_GetVgaCcmLin3VgaFr01NoResp(Channel)  InputArgumentSpecifications=VGAChlType(0)  OutputArgumentSpecifications=uint8(1), P_Std_ReturnType
  MirrorLin3ErrServ_GetVgaRxErrSta : FunctionCaller  FunctionPrototype=[ErrFlag,ERR] = MirrorLin3ErrServ_GetVgaRxErrSta(Channel)  InputArgumentSpecifications=VGAChlType(0)  OutputArgumentSpecifications=uint8(1), P_Std_ReturnType
  MirrorLinFrameServ_GetRespSta : FunctionCaller  FunctionPrototype=[RxAckSts,RxData,ERR] = MirrorLinFrameServ_GetRespSta(FrameId)  InputArgumentSpecifications=LinFrameType(0)  OutputArgumentSpecifications=P_LinAckType, P_LinDataType, P_Std_ReturnType
  NvMServ_HfaMtrCalReadBlock : FunctionCaller  FunctionPrototype=[Data,ERR] = NvMServ_HfaMtrCalReadBlock()  OutputArgumentSpecifications=uint8([1]), P_Std_ReturnType
  NvMServ_HfaMtrCalWriteBlock : FunctionCaller  FunctionPrototype=ERR = NvMServ_HfaMtrCalWriteBlock(Data)  InputArgumentSpecifications=uint8([1])  OutputArgumentSpecifications=P_Std_ReturnType
  NvMServ_NvMNotifyJobWorking : FunctionCaller  FunctionPrototype=[Status,ERR] = NvMServ_NvMNotifyJobWorking(BlockId)  InputArgumentSpecifications=NvMBlockId(0)  OutputArgumentSpecifications=uint8(1), P_Std_ReturnType
  NvMServ_VgaMtrCalReadBlock : FunctionCaller  FunctionPrototype=[Data,ERR] = NvMServ_VgaMtrCalReadBlock()  OutputArgumentSpecifications=uint8([1]), P_Std_ReturnType
  NvMServ_VgaMtrCalWriteBlock : FunctionCaller  FunctionPrototype=ERR = NvMServ_VgaMtrCalWriteBlock(Data)  InputArgumentSpecifications=uint8([1])  OutputArgumentSpecifications=P_Std_ReturnType
  NvMServ_WriteBlock : FunctionCaller  FunctionPrototype=ERR = NvMServ_WriteBlock(BlockId,Data)  InputArgumentSpecifications=NvMBlockId(0), uint8([1])  OutputArgumentSpecifications=P_Std_ReturnType
  PA_NvMBlockVgaMtrCalDataInit : Constant  Value=NvMBlockVgaMtrCalDataInit
  PwrParaService_GetMotorCailCnt : FunctionCaller  FunctionPrototype=[Cnt,ERR] = PwrParaService_GetMotorCailCnt(Channel)  InputArgumentSpecifications=PowerChlType(0)  OutputArgumentSpecifications=uint8(1), P_Std_ReturnType
  PwrParaService_GetMotorCailNum : FunctionCaller  FunctionPrototype=[Num,ERR] = PwrParaService_GetMotorCailNum(Channel)  InputArgumentSpecifications=PowerChlType(0)  OutputArgumentSpecifications=uint8(1), P_Std_ReturnType
  PwrParaService_SetMotorCailCnt : FunctionCaller  FunctionPrototype=ERR = PwrParaService_SetMotorCailCnt(Channel,Cnt)  InputArgumentSpecifications=PowerChlType(0), uint8(1)  OutputArgumentSpecifications=P_Std_ReturnType
  PwrParaService_SetMotorCailNum : FunctionCaller  FunctionPrototype=ERR = PwrParaService_SetMotorCailNum(Channel,Num)  InputArgumentSpecifications=PowerChlType(0), uint8(1)  OutputArgumentSpecifications=P_Std_ReturnType
  Terminator_{1..549} (549 alike) : Terminator
  VentnMotAdr_VentnMotAdr_write : Outport
      settings: EnsureOutportIsVirtual=on
  VentnMotAdr2_VentnMotAdr2_write : Outport  Port=2
      settings: EnsureOutportIsVirtual=on
  VentnMotBlockCmd_VentnMotBlockCmd_write : Outport  Port=3
      settings: EnsureOutportIsVirtual=on
  VentnMotBlockCmd2_VentnMotBlockCmd2_write : Outport  Port=4
      settings: EnsureOutportIsVirtual=on
  VentnMotClearReq_VentnMotClearReq_write : Outport  Port=5
      settings: EnsureOutportIsVirtual=on
  VentnMotClearReq2_VentnMotClearReq2_write : Outport  Port=6
      settings: EnsureOutportIsVirtual=on
  VentnMotCoilPwrOn_VentnMotCoilPwrOn_write : Outport  Port=7
      settings: EnsureOutportIsVirtual=on
  VentnMotCoilPwrOn2_VentnMotCoilPwrOn2_write : Outport  Port=8
      settings: EnsureOutportIsVirtual=on
  VentnMotDir_VentnMotDir_write : Outport  Port=9
      settings: EnsureOutportIsVirtual=on
  VentnMotDir2_VentnMotDir2_write : Outport  Port=10
      settings: EnsureOutportIsVirtual=on
  VentnMotMode_VentnMotMode_write : Outport  Port=11
      settings: EnsureOutportIsVirtual=on
  VentnMotMode2_VentnMotMode2_write : Outport  Port=12
      settings: EnsureOutportIsVirtual=on
  VentnMotPsonAvl_VentnMotPsonAvl_write : Outport  Port=13
      settings: EnsureOutportIsVirtual=on
  VentnMotPsonAvl2_VentnMotPsonAvl2_write : Outport  Port=14